simulink model "SdbTxSigProc"  (R2022b)
settings: Solver=FixedStepDiscrete  StartTime=0.0  StopTime=10.0  FixedStep=0.1  RelTol=1e-3
(part 4 of 4)

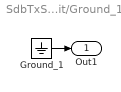
== system SdbTxSigProc/SdbTxSigProc_Init/Ground_1 ==
  Ground_1 : Ground
  Out1 : Outport  OutDataTypeStr=Bus: UInt64Rec  PortDimensions=1
  wires:
  Ground_1/1 -> Out1/1

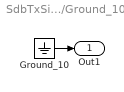
== system SdbTxSigProc/SdbTxSigProc_Init/Ground_10 ==
  Ground_10 : Ground
  Out1 : Outport  OutDataTypeStr=Bus: UInt64Rec  PortDimensions=1
  wires:
  Ground_10/1 -> Out1/1

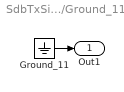
== system SdbTxSigProc/SdbTxSigProc_Init/Ground_11 ==
  Ground_11 : Ground
  Out1 : Outport  OutDataTypeStr=Bus: UInt64Rec  PortDimensions=1
  wires:
  Ground_11/1 -> Out1/1

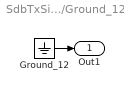
== system SdbTxSigProc/SdbTxSigProc_Init/Ground_12 ==
  Ground_12 : Ground
  Out1 : Outport  OutDataTypeStr=Bus: UInt64Rec  PortDimensions=1
  wires:
  Ground_12/1 -> Out1/1

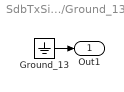
== system SdbTxSigProc/SdbTxSigProc_Init/Ground_13 ==
  Ground_13 : Ground
  Out1 : Outport  OutDataTypeStr=Bus: UInt64Rec  PortDimensions=1
  wires:
  Ground_13/1 -> Out1/1

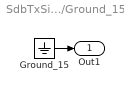
== system SdbTxSigProc/SdbTxSigProc_Init/Ground_15 ==
  Ground_15 : Ground
  Out1 : Outport  OutDataTypeStr=Bus: CmptmtAirTEstimdExtd  PortDimensions=1
  wires:
  Ground_15/1 -> Out1/1

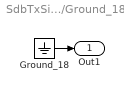
== system SdbTxSigProc/SdbTxSigProc_Init/Ground_18 ==
  Ground_18 : Ground
  Out1 : Outport  OutDataTypeStr=Bus: EvaprTFrnt  PortDimensions=1
  wires:
  Ground_18/1 -> Out1/1

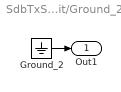
== system SdbTxSigProc/SdbTxSigProc_Init/Ground_2 ==
  Ground_2 : Ground
  Out1 : Outport  OutDataTypeStr=Bus: UInt64Rec  PortDimensions=1
  wires:
  Ground_2/1 -> Out1/1

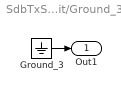
== system SdbTxSigProc/SdbTxSigProc_Init/Ground_3 ==
  Ground_3 : Ground
  Out1 : Outport  OutDataTypeStr=Bus: UInt64Rec  PortDimensions=1
  wires:
  Ground_3/1 -> Out1/1

== system SdbTxSigProc/SdbTxSigProc_Init/Ground_4 ==
  Ground_4 : Ground
  Out1 : Outport  OutDataTypeStr=Bus: UInt64Rec  PortDimensions=1
  wires:
  Ground_4/1 -> Out1/1

== system SdbTxSigProc/SdbTxSigProc_Init/Ground_5 ==
  Ground_5 : Ground
  Out1 : Outport  OutDataTypeStr=Bus: UInt64Rec  PortDimensions=1
  wires:
  Ground_5/1 -> Out1/1

== system SdbTxSigProc/SdbTxSigProc_Init/Ground_6 ==
  Ground_6 : Ground
  Out1 : Outport  OutDataTypeStr=Bus: UInt64Rec  PortDimensions=1
  wires:
  Ground_6/1 -> Out1/1

== system SdbTxSigProc/SdbTxSigProc_Init/Ground_7 ==
  Ground_7 : Ground
  Out1 : Outport  OutDataTypeStr=Bus: UInt64Rec  PortDimensions=1
  wires:
  Ground_7/1 -> Out1/1

== system SdbTxSigProc/SdbTxSigProc_Init/Ground_8 ==
  Ground_8 : Ground
  Out1 : Outport  OutDataTypeStr=Bus: AmbTEstimd  PortDimensions=1
  wires:
  Ground_8/1 -> Out1/1

== system SdbTxSigProc/SdbTxSigProc_Init/Ground_9 ==
  Ground_9 : Ground
  Out1 : Outport  OutDataTypeStr=Bus: UInt64Rec  PortDimensions=1
  wires:
  Ground_9/1 -> Out1/1

== system SdbTxSigProc/SdbTxSigProc_Runnable_sys/Ground_10 ==
  Ground_10 : Ground
  Out1 : Outport  OutDataTypeStr=Bus: Develp1  PortDimensions=1
  wires:
  Ground_10/1 -> Out1/1

== system SdbTxSigProc/SdbTxSigProc_Runnable_sys/Ground_11 ==
  Ground_11 : Ground
  Out1 : Outport  OutDataTypeStr=Bus: Develp1  PortDimensions=1
  wires:
  Ground_11/1 -> Out1/1

== system SdbTxSigProc/SdbTxSigProc_Runnable_sys/Ground_117 ==
  Ground_117 : Ground
  Out1 : Outport  OutDataTypeStr=Bus: AirTFbFromClimaRec  PortDimensions=1
  wires:
  Ground_117/1 -> Out1/1

== system SdbTxSigProc/SdbTxSigProc_Runnable_sys/Ground_118 ==
  Ground_118 : Ground
  Out1 : Outport  OutDataTypeStr=Bus: AmbTEstimd  PortDimensions=1
  wires:
  Ground_118/1 -> Out1/1

== system SdbTxSigProc/SdbTxSigProc_Runnable_sys/Ground_12 ==
  Ground_12 : Ground
  Out1 : Outport  OutDataTypeStr=Bus: Develp1  PortDimensions=1
  wires:
  Ground_12/1 -> Out1/1

== system SdbTxSigProc/SdbTxSigProc_Runnable_sys/Ground_120 ==
  Ground_120 : Ground
  Out1 : Outport  OutDataTypeStr=Bus: UInt64Rec  PortDimensions=1
  wires:
  Ground_120/1 -> Out1/1

== system SdbTxSigProc/SdbTxSigProc_Runnable_sys/Ground_121 ==
  Ground_121 : Ground
  Out1 : Outport  OutDataTypeStr=Bus: UInt64Rec  PortDimensions=1
  wires:
  Ground_121/1 -> Out1/1

== system SdbTxSigProc/SdbTxSigProc_Runnable_sys/Ground_122 ==
  Ground_122 : Ground
  Out1 : Outport  OutDataTypeStr=Bus: UInt64Rec  PortDimensions=1
  wires:
  Ground_122/1 -> Out1/1

== system SdbTxSigProc/SdbTxSigProc_Runnable_sys/Ground_123 ==
  Ground_123 : Ground
  Out1 : Outport  OutDataTypeStr=Bus: UInt64Rec  PortDimensions=1
  wires:
  Ground_123/1 -> Out1/1

== system SdbTxSigProc/SdbTxSigProc_Runnable_sys/Ground_124 ==
  Ground_124 : Ground
  Out1 : Outport  OutDataTypeStr=Bus: UInt64Rec  PortDimensions=1
  wires:
  Ground_124/1 -> Out1/1

== system SdbTxSigProc/SdbTxSigProc_Runnable_sys/Ground_125 ==
  Ground_125 : Ground
  Out1 : Outport  OutDataTypeStr=Bus: UInt64Rec  PortDimensions=1
  wires:
  Ground_125/1 -> Out1/1

== system SdbTxSigProc/SdbTxSigProc_Runnable_sys/Ground_126 ==
  Ground_126 : Ground
  Out1 : Outport  OutDataTypeStr=Bus: UInt64Rec  PortDimensions=1
  wires:
  Ground_126/1 -> Out1/1

== system SdbTxSigProc/SdbTxSigProc_Runnable_sys/Ground_127 ==
  Ground_127 : Ground
  Out1 : Outport  OutDataTypeStr=Bus: UInt64Rec  PortDimensions=1
  wires:
  Ground_127/1 -> Out1/1

== system SdbTxSigProc/SdbTxSigProc_Runnable_sys/Ground_128 ==
  Ground_128 : Ground
  Out1 : Outport  OutDataTypeStr=Bus: UInt64Rec  PortDimensions=1
  wires:
  Ground_128/1 -> Out1/1

== system SdbTxSigProc/SdbTxSigProc_Runnable_sys/Ground_129 ==
  Ground_129 : Ground
  Out1 : Outport  OutDataTypeStr=Bus: UInt64Rec  PortDimensions=1
  wires:
  Ground_129/1 -> Out1/1

== system SdbTxSigProc/SdbTxSigProc_Runnable_sys/Ground_130 ==
  Ground_130 : Ground
  Out1 : Outport  OutDataTypeStr=Bus: UInt64Rec  PortDimensions=1
  wires:
  Ground_130/1 -> Out1/1

== system SdbTxSigProc/SdbTxSigProc_Runnable_sys/Ground_131 ==
  Ground_131 : Ground
  Out1 : Outport  OutDataTypeStr=Bus: UInt64Rec  PortDimensions=1
  wires:
  Ground_131/1 -> Out1/1

== system SdbTxSigProc/SdbTxSigProc_Runnable_sys/Ground_143 ==
  Ground_143 : Ground
  Out1 : Outport  OutDataTypeStr=Bus: CmptmtAirTEstimdExtd  PortDimensions=1
  wires:
  Ground_143/1 -> Out1/1

== system SdbTxSigProc/SdbTxSigProc_Runnable_sys/Ground_150 ==
  Ground_150 : Ground
  Out1 : Outport  OutDataTypeStr=Bus: DefrstReq  PortDimensions=1
  wires:
  Ground_150/1 -> Out1/1

== system SdbTxSigProc/SdbTxSigProc_Runnable_sys/Ground_153 ==
  Ground_153 : Ground
  Out1 : Outport  OutDataTypeStr=Bus: EvaprTFrnt  PortDimensions=1
  wires:
  Ground_153/1 -> Out1/1

== system SdbTxSigProc/SdbTxSigProc_Runnable_sys/Ground_159 ==
  Ground_159 : Ground
  Out1 : Outport  OutDataTypeStr=Bus: EvaprTFrnt  PortDimensions=1
  wires:
  Ground_159/1 -> Out1/1

== system SdbTxSigProc/SdbTxSigProc_Runnable_sys/Ground_160 ==
  Ground_160 : Ground
  Out1 : Outport  OutDataTypeStr=Bus: EvaprTFrnt  PortDimensions=1
  wires:
  Ground_160/1 -> Out1/1

== system SdbTxSigProc/SdbTxSigProc_Runnable_sys/Ground_161 ==
  Ground_161 : Ground
  Out1 : Outport  OutDataTypeStr=Bus: EvaprTFrnt  PortDimensions=1
  wires:
  Ground_161/1 -> Out1/1

== system SdbTxSigProc/SdbTxSigProc_Runnable_sys/Ground_162 ==
  Ground_162 : Ground
  Out1 : Outport  OutDataTypeStr=Bus: EvaprTFrnt  PortDimensions=1
  wires:
  Ground_162/1 -> Out1/1

== system SdbTxSigProc/SdbTxSigProc_Runnable_sys/Ground_163 ==
  Ground_163 : Ground
  Out1 : Outport  OutDataTypeStr=Bus: EvaprTFrnt  PortDimensions=1
  wires:
  Ground_163/1 -> Out1/1

== system SdbTxSigProc/SdbTxSigProc_Runnable_sys/Ground_164 ==
  Ground_164 : Ground
  Out1 : Outport  OutDataTypeStr=Bus: EvaprTFrnt  PortDimensions=1
  wires:
  Ground_164/1 -> Out1/1

== system SdbTxSigProc/SdbTxSigProc_Runnable_sys/Ground_165 ==
  Ground_165 : Ground
  Out1 : Outport  OutDataTypeStr=Bus: EvaprTFrnt  PortDimensions=1
  wires:
  Ground_165/1 -> Out1/1

== system SdbTxSigProc/SdbTxSigProc_Runnable_sys/Ground_166 ==
  Ground_166 : Ground
  Out1 : Outport  OutDataTypeStr=Bus: EvaprTFrnt  PortDimensions=1
  wires:
  Ground_166/1 -> Out1/1

== system SdbTxSigProc/SdbTxSigProc_Runnable_sys/Ground_167 ==
  Ground_167 : Ground
  Out1 : Outport  OutDataTypeStr=Bus: EvaprTFrnt  PortDimensions=1
  wires:
  Ground_167/1 -> Out1/1

== system SdbTxSigProc/SdbTxSigProc_Runnable_sys/Ground_173 ==
  Ground_173 : Ground
  Out1 : Outport  OutDataTypeStr=Bus: EvaprTFrnt  PortDimensions=1
  wires:
  Ground_173/1 -> Out1/1

== system SdbTxSigProc/SdbTxSigProc_Runnable_sys/Ground_196 ==
  Ground_196 : Ground
  Out1 : Outport  OutDataTypeStr=Bus: AirQlyMeasInValReq  PortDimensions=1
  wires:
  Ground_196/1 -> Out1/1

== system SdbTxSigProc/SdbTxSigProc_Runnable_sys/Ground_36 ==
  Ground_36 : Ground
  Out1 : Outport  OutDataTypeStr=Bus: CmptmtAirDistbnRsp  PortDimensions=1
  wires:
  Ground_36/1 -> Out1/1

== system SdbTxSigProc/SdbTxSigProc_Runnable_sys/Ground_50 ==
  Ground_50 : Ground
  Out1 : Outport  OutDataTypeStr=Bus: EvaprTFrnt  PortDimensions=1
  wires:
  Ground_50/1 -> Out1/1

== system SdbTxSigProc/SdbTxSigProc_Runnable_sys/Ground_51 ==
  Ground_51 : Ground
  Out1 : Outport  OutDataTypeStr=Bus: EvaprTFrnt  PortDimensions=1
  wires:
  Ground_51/1 -> Out1/1

== system SdbTxSigProc/SdbTxSigProc_Runnable_sys/Ground_58 ==
  Ground_58 : Ground
  Out1 : Outport  OutDataTypeStr=Bus: HvacAirTForHeatrFrnt  PortDimensions=1
  wires:
  Ground_58/1 -> Out1/1

== system SdbTxSigProc/SdbTxSigProc_Runnable_sys/Ground_59 ==
  Ground_59 : Ground
  Out1 : Outport  OutDataTypeStr=Bus: HvacAirTForHeatrFrnt  PortDimensions=1
  wires:
  Ground_59/1 -> Out1/1

== system SdbTxSigProc/SdbTxSigProc_Runnable_sys/Ground_6 ==
  Ground_6 : Ground
  Out1 : Outport  OutDataTypeStr=Bus: Develp1  PortDimensions=1
  wires:
  Ground_6/1 -> Out1/1

== system SdbTxSigProc/SdbTxSigProc_Runnable_sys/Ground_60 ==
  Ground_60 : Ground
  Out1 : Outport  OutDataTypeStr=Bus: HvacAirTForHeatrFrnt  PortDimensions=1
  wires:
  Ground_60/1 -> Out1/1

== system SdbTxSigProc/SdbTxSigProc_Runnable_sys/Ground_64 ==
  Ground_64 : Ground
  Out1 : Outport  OutDataTypeStr=Bus: RemSynchrnztnLcl  PortDimensions=1
  wires:
  Ground_64/1 -> Out1/1

== system SdbTxSigProc/SdbTxSigProc_Runnable_sys/Ground_7 ==
  Ground_7 : Ground
  Out1 : Outport  OutDataTypeStr=Bus: Develp1  PortDimensions=1
  wires:
  Ground_7/1 -> Out1/1

== system SdbTxSigProc/SdbTxSigProc_Runnable_sys/Ground_8 ==
  Ground_8 : Ground
  Out1 : Outport  OutDataTypeStr=Bus: Develp1  PortDimensions=1
  wires:
  Ground_8/1 -> Out1/1

== system SdbTxSigProc/SdbTxSigProc_Runnable_sys/Ground_80 ==
  Ground_80 : Ground
  Out1 : Outport  OutDataTypeStr=Bus: DefrstReq  PortDimensions=1
  wires:
  Ground_80/1 -> Out1/1

== system SdbTxSigProc/SdbTxSigProc_Runnable_sys/Ground_9 ==
  Ground_9 : Ground
  Out1 : Outport  OutDataTypeStr=Bus: Develp1  PortDimensions=1
  wires:
  Ground_9/1 -> Out1/1

== system SdbTxSigProc/SdbTxSigProc_Runnable_sys/Ground_92 ==
  Ground_92 : Ground
  Out1 : Outport  OutDataTypeStr=Bus: Boolean32  PortDimensions=1
  wires:
  Ground_92/1 -> Out1/1
